simulink model "CCMAdcM"  (R2022b)
settings: Solver=FixedStepDiscrete  StartTime=0.0  StopTime=10.0  FixedStep=0.01  RelTol=1e-3
settings Model: Creator=blue.ge  LastModifiedBy=blue.ge
settings DataIOCC: SaveFormat=StructureWithTime
settings OptimizationCC: DefaultParameterBehavior=Inlined  EnhancedBackFolding=on  PassReuseOutputArgsAs=Individual arguments  ZeroExternalMemoryAtStartup=off  ZeroInternalMemoryAtStartup=off  OptimizeBlockOrder=speed  DifferentSizesBufferReuse=on  OptimizationCustomize=off  EfficientTunableParamExpr=on
settings DebuggingCC: ParameterTunabilityLossMsg=error
settings ModelReferenceCC: ModelReferenceNumInstancesAllowed=Single
settings SFSimCC: MATLABDynamicMemAlloc=off
settings RTWCC: Description=AUTOSAR  SystemTargetFile=autosar.tlc  GenCodeOnly=on  TemplateMakefile=ert_default_tmf
settings CodeAppCC: ForceParamTrailComments=on  IgnoreCustomStorageClasses=off  OperatorAnnotations=on  SimulinkDataObjDesc=on  SFDataObjDesc=on  InsertBlockDesc=on
settings STFCustomTargetCC: TargetLangStandard=C99 (ISO)  MultiwordLength=256  ParMdlRefBuildCompliant=on  CompOptLevelCompliant=on  IncludeMdlTerminateFcn=off  CombineOutputUpdateFcns=on  SuppressErrorStatus=on  ERTCustomFileBanners=on  SupportNonFinite=off  SupportComplex=off  SupportContinuousTime=off  SupportNonInlinedSFcns=off  RemoveResetFunc=on  MATLABClassNameForMDSCustomization=Simulink.SoftwareTarget.ERTCustomization  AutosarCompliant=on  UseToolchainInfoCompliant=on  LookupTableObjectStructAxisOrder=2,1,3,4,...  LUTObjectStructOrderEvenSpacing=Size,Table,Breakpoints  SystemTargetFile=autosar.tlc
settings ERTTargetCC: TargetLangStandard=C99 (ISO)  ConcurrentExecutionCompliant=off  IncludeMdlTerminateFcn=off  SuppressErrorStatus=on  SupportNonFinite=off  SupportComplex=off  ModelStepFunctionPrototypeControlCompliant=off  CPPClassGenCompliant=off  AutosarCompliant=on  LookupTableObjectStructAxisOrder=2,1,3,4,...  LUTObjectStructOrderEvenSpacing=Size,Table,Breakpoints  InlinedParameterPlacement=NonHierarchical  ERTCustomFileTemplate=example_file_process.tlc

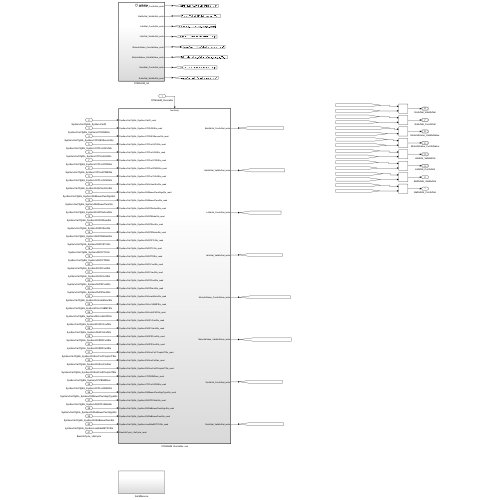
[thumbnail: Simulink's stored preview of the top-level diagram]
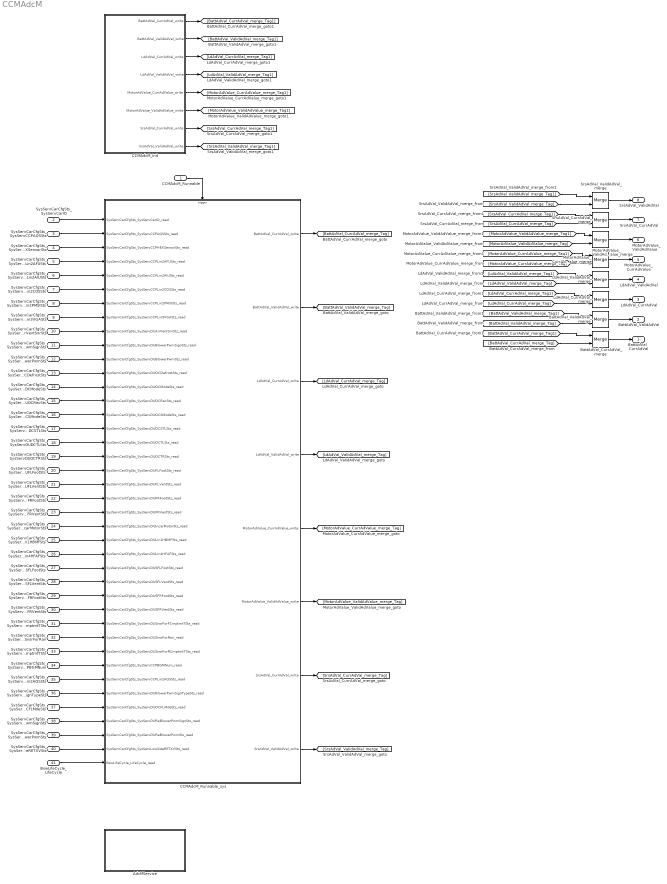
== system CCMAdcM ==
  CCMAdcM_Runnable : Inport  SampleTime=0.01  OutputFunctionCall=on
  SysServCarCfgSts_SysServCarID : Inport  Port=2  OutDataTypeStr=Enum: SysServCarID  PortDimensions=1
  SysServCarCfgSts_SysServCCPAQSSts : Inport  Port=3  OutDataTypeStr=UInt8  PortDimensions=1
  SysServCarCfgSts_SysServCCPHEXSensorSts : Inport  Port=4  OutDataTypeStr=UInt8  PortDimensions=1
  SysServCarCfgSts_SysServCCPLin2AFUSts : Inport  Port=5  OutDataTypeStr=Boolean  PortDimensions=1
  SysServCarCfgSts_SysServCCPLin2AIUSts : Inport  Port=6  OutDataTypeStr=UInt8  PortDimensions=1
  SysServCarCfgSts_SysServCCPLin2CDSSts : Inport  Port=7  OutDataTypeStr=Boolean  PortDimensions=1
  SysServCarCfgSts_SysServCCPLin2PMSISts : Inport  Port=8  OutDataTypeStr=Boolean  PortDimensions=1
  SysServCarCfgSts_SysServCCPLin3VGASts : Inport  Port=9  OutDataTypeStr=Boolean  PortDimensions=1
  SysServCarCfgSts_SysServDUAirVentSnrSts : Inport  Port=10  OutDataTypeStr=Boolean  PortDimensions=1
  SysServCarCfgSts_SysServDUBlowerPwmSignSts : Inport  Port=11  OutDataTypeStr=Boolean  PortDimensions=1
  SysServCarCfgSts_SysServDUBlowerPwmSts : Inport  Port=12  OutDataTypeStr=Boolean  PortDimensions=1
  SysServCarCfgSts_SysServDUDCDefrostSts : Inport  Port=13  OutDataTypeStr=UInt8  PortDimensions=1
  SysServCarCfgSts_SysServDUDCModeSts : Inport  Port=14  OutDataTypeStr=UInt8  PortDimensions=1
  SysServCarCfgSts_SysServDUDCRecSts : Inport  Port=15  OutDataTypeStr=UInt8  PortDimensions=1
  SysServCarCfgSts_SysServDUDCSModeSts : Inport  Port=16  OutDataTypeStr=UInt8  PortDimensions=1
  SysServCarCfgSts_SysServDUDCSTLSts : Inport  Port=17  OutDataTypeStr=UInt8  PortDimensions=1
  SysServCarCfgSts_SysServDUDCTLSts : Inport  Port=18  OutDataTypeStr=UInt8  PortDimensions=1
  SysServCarCfgSts_SysServDUDCTRSts : Inport  Port=19  OutDataTypeStr=UInt8  PortDimensions=1
  SysServCarCfgSts_SysServDUFLFootSts : Inport  Port=20  OutDataTypeStr=UInt8  PortDimensions=1
  SysServCarCfgSts_SysServDUFLVentSts : Inport  Port=21  OutDataTypeStr=UInt8  PortDimensions=1
  SysServCarCfgSts_SysServDUFRFootSts : Inport  Port=22  OutDataTypeStr=UInt8  PortDimensions=1
  SysServCarCfgSts_SysServDUFRVentSts : Inport  Port=23  OutDataTypeStr=UInt8  PortDimensions=1
  SysServCarCfgSts_SysServDUIncarMotorSts : Inport  Port=24  OutDataTypeStr=Boolean  PortDimensions=1
  SysServCarCfgSts_SysServDULin1HBMFSts : Inport  Port=25  OutDataTypeStr=Boolean  PortDimensions=1
  SysServCarCfgSts_SysServDULin4HFAFSts : Inport  Port=26  OutDataTypeStr=UInt32  PortDimensions=1
  SysServCarCfgSts_SysServDUSFLFootSts : Inport  Port=27  OutDataTypeStr=UInt8  PortDimensions=1
  SysServCarCfgSts_SysServDUSFLVentSts : Inport  Port=28  OutDataTypeStr=UInt8  PortDimensions=1
  SysServCarCfgSts_SysServDUSFRFootSts : Inport  Port=29  OutDataTypeStr=UInt8  PortDimensions=1
  SysServCarCfgSts_SysServDUSFRVentSts : Inport  Port=30  OutDataTypeStr=UInt8  PortDimensions=1
  SysServCarCfgSts_SysServDUSnsrForFCmptmtTSts : Inport  Port=31  OutDataTypeStr=UInt8  PortDimensions=1
  SysServCarCfgSts_SysServDUSnsrForRair : Inport  Port=32  OutDataTypeStr=UInt8  PortDimensions=1
  SysServCarCfgSts_SysServDUSnsrForRCmptmtTSts : Inport  Port=33  OutDataTypeStr=UInt8  PortDimensions=1
  SysServCarCfgSts_SysServCCPBGMNum : Inport  Port=34  OutDataTypeStr=UInt8  PortDimensions=1
  SysServCarCfgSts_SysServCCPLin2AQSSts : Inport  Port=35  OutDataTypeStr=Boolean  PortDimensions=1
  SysServCarCfgSts_SysServDUBlowerPwmSignTypeSts : Inport  Port=36  OutDataTypeStr=UInt8  PortDimensions=1
  SysServCarCfgSts_SysServDUDCFLMdeSts : Inport  Port=37  OutDataTypeStr=UInt8  PortDimensions=1
  SysServCarCfgSts_SysServDUReBlowerPwmSignSts : Inport  Port=38  OutDataTypeStr=UInt8  PortDimensions=1
  SysServCarCfgSts_SysServDUReBlowerPwmSts : Inport  Port=39  OutDataTypeStr=UInt8  PortDimensions=1
  SysServCarCfgSts_SysServLowSideRETXVSts : Inport  Port=40  OutDataTypeStr=UInt8  PortDimensions=1
  BswLifeCycle_LifeCycle : Inport  Port=41  OutDataTypeStr=Enum: CCMLifeCycleModeGroup  PortDimensions=1
  AdcMService : Subsystem
  BattAdVal_CurrAdVal_merge : Merge
  BattAdVal_ValidAdVal_merge : Merge
  CCMAdcM_Init : Subsystem
  CCMAdcM_Runnable_sys : Subsystem
  LdAdVal_CurrAdVal_merge : Merge
  LdAdVal_ValidAdVal_merge : Merge
  MotorAdValue_CurrAdValue_merge : Merge
  MotorAdValue_ValidAdValue_merge : Merge
  SrsAdVal_CurrAdVal_merge : Merge
  SrsAdVal_ValidAdVal_merge : Merge
  BattAdVal_CurrAdVal : Outport  OutDataTypeStr=BatCurrAd  PortDimensions=1
  BattAdVal_ValidAdVal : Outport  Port=2  OutDataTypeStr=BatValidAd  PortDimensions=1
  LdAdVal_CurrAdVal : Outport  Port=3  OutDataTypeStr=Bus: LdAd  PortDimensions=1  BusOutputAsStruct=on
  LdAdVal_ValidAdVal : Outport  Port=4  OutDataTypeStr=Bus: LdAd  PortDimensions=1  BusOutputAsStruct=on
  MotorAdValue_CurrAdValue : Outport  Port=5  OutDataTypeStr=Bus: MotorAdValueType  PortDimensions=1  BusOutputAsStruct=on
  MotorAdValue_ValidAdValue : Outport  Port=6  OutDataTypeStr=Bus: MotorAdValueType  PortDimensions=1  BusOutputAsStruct=on
  SrsAdVal_CurrAdVal : Outport  Port=7  OutDataTypeStr=Bus: SensorAd  PortDimensions=1  BusOutputAsStruct=on
  SrsAdVal_ValidAdVal : Outport  Port=8  OutDataTypeStr=Bus: SensorAd  PortDimensions=1  BusOutputAsStruct=on
  wires:
  SysServCarCfgSts_SysServCarID/1 -> CCMAdcM_Runnable_sys/1(SysServCarCfgSts_SysServCarID_read)
  SysServCarCfgSts_SysServCCPAQSSts/1 -> CCMAdcM_Runnable_sys/2(SysServCarCfgSts_SysServCCPAQSSts_read)
  SysServCarCfgSts_SysServCCPHEXSensorSts/1 -> CCMAdcM_Runnable_sys/3(SysServCarCfgSts_SysServCCPHEXSensorSts_read)
  SysServCarCfgSts_SysServCCPLin2AFUSts/1 -> CCMAdcM_Runnable_sys/4(SysServCarCfgSts_SysServCCPLin2AFUSts_read)
  SysServCarCfgSts_SysServCCPLin2AIUSts/1 -> CCMAdcM_Runnable_sys/5(SysServCarCfgSts_SysServCCPLin2AIUSts_read)
  SysServCarCfgSts_SysServCCPLin2CDSSts/1 -> CCMAdcM_Runnable_sys/6(SysServCarCfgSts_SysServCCPLin2CDSSts_read)
  SysServCarCfgSts_SysServCCPLin2PMSISts/1 -> CCMAdcM_Runnable_sys/7(SysServCarCfgSts_SysServCCPLin2PMSISts_read)
  SysServCarCfgSts_SysServCCPLin3VGASts/1 -> CCMAdcM_Runnable_sys/8(SysServCarCfgSts_SysServCCPLin3VGASts_read)
  SysServCarCfgSts_SysServDUAirVentSnrSts/1 -> CCMAdcM_Runnable_sys/9(SysServCarCfgSts_SysServDUAirVentSnrSts_read)
  SysServCarCfgSts_SysServDUBlowerPwmSignSts/1 -> CCMAdcM_Runnable_sys/10(SysServCarCfgSts_SysServDUBlowerPwmSignSts_read)
  SysServCarCfgSts_SysServDUBlowerPwmSts/1 -> CCMAdcM_Runnable_sys/11(SysServCarCfgSts_SysServDUBlowerPwmSts_read)
  SysServCarCfgSts_SysServDUDCDefrostSts/1 -> CCMAdcM_Runnable_sys/12(SysServCarCfgSts_SysServDUDCDefrostSts_read)
  SysServCarCfgSts_SysServDUDCModeSts/1 -> CCMAdcM_Runnable_sys/13(SysServCarCfgSts_SysServDUDCModeSts_read)
  SysServCarCfgSts_SysServDUDCRecSts/1 -> CCMAdcM_Runnable_sys/14(SysServCarCfgSts_SysServDUDCRecSts_read)
  SysServCarCfgSts_SysServDUDCSModeSts/1 -> CCMAdcM_Runnable_sys/15(SysServCarCfgSts_SysServDUDCSModeSts_read)
  SysServCarCfgSts_SysServDUDCSTLSts/1 -> CCMAdcM_Runnable_sys/16(SysServCarCfgSts_SysServDUDCSTLSts_read)
  SysServCarCfgSts_SysServDUDCTLSts/1 -> CCMAdcM_Runnable_sys/17(SysServCarCfgSts_SysServDUDCTLSts_read)
  SysServCarCfgSts_SysServDUDCTRSts/1 -> CCMAdcM_Runnable_sys/18(SysServCarCfgSts_SysServDUDCTRSts_read)
  SysServCarCfgSts_SysServDUFLFootSts/1 -> CCMAdcM_Runnable_sys/19(SysServCarCfgSts_SysServDUFLFootSts_read)
  SysServCarCfgSts_SysServDUFLVentSts/1 -> CCMAdcM_Runnable_sys/20(SysServCarCfgSts_SysServDUFLVentSts_read)
  SysServCarCfgSts_SysServDUFRFootSts/1 -> CCMAdcM_Runnable_sys/21(SysServCarCfgSts_SysServDUFRFootSts_read)
  SysServCarCfgSts_SysServDUFRVentSts/1 -> CCMAdcM_Runnable_sys/22(SysServCarCfgSts_SysServDUFRVentSts_read)
  SysServCarCfgSts_SysServDUIncarMotorSts/1 -> CCMAdcM_Runnable_sys/23(SysServCarCfgSts_SysServDUIncarMotorSts_read)
  SysServCarCfgSts_SysServDULin1HBMFSts/1 -> CCMAdcM_Runnable_sys/24(SysServCarCfgSts_SysServDULin1HBMFSts_read)
  SysServCarCfgSts_SysServDULin4HFAFSts/1 -> CCMAdcM_Runnable_sys/25(SysServCarCfgSts_SysServDULin4HFAFSts_read)
  SysServCarCfgSts_SysServDUSFLFootSts/1 -> CCMAdcM_Runnable_sys/26(SysServCarCfgSts_SysServDUSFLFootSts_read)
  SysServCarCfgSts_SysServDUSFLVentSts/1 -> CCMAdcM_Runnable_sys/27(SysServCarCfgSts_SysServDUSFLVentSts_read)
  SysServCarCfgSts_SysServDUSFRFootSts/1 -> CCMAdcM_Runnable_sys/28(SysServCarCfgSts_SysServDUSFRFootSts_read)
  SysServCarCfgSts_SysServDUSFRVentSts/1 -> CCMAdcM_Runnable_sys/29(SysServCarCfgSts_SysServDUSFRVentSts_read)
  SysServCarCfgSts_SysServDUSnsrForFCmptmtTSts/1 -> CCMAdcM_Runnable_sys/30(SysServCarCfgSts_SysServDUSnsrForFCmptmtTSts_read)
  SysServCarCfgSts_SysServDUSnsrForRair/1 -> CCMAdcM_Runnable_sys/31(SysServCarCfgSts_SysServDUSnsrForRair_read)
  SysServCarCfgSts_SysServDUSnsrForRCmptmtTSts/1 -> CCMAdcM_Runnable_sys/32(SysServCarCfgSts_SysServDUSnsrForRCmptmtTSts_read)
  SysServCarCfgSts_SysServCCPBGMNum/1 -> CCMAdcM_Runnable_sys/33(SysServCarCfgSts_SysServCCPBGMNum_read)
  SysServCarCfgSts_SysServCCPLin2AQSSts/1 -> CCMAdcM_Runnable_sys/34(SysServCarCfgSts_SysServCCPLin2AQSSts_read)
  SysServCarCfgSts_SysServDUBlowerPwmSignTypeSts/1 -> CCMAdcM_Runnable_sys/35(SysServCarCfgSts_SysServDUBlowerPwmSignTypeSts_read)
  SysServCarCfgSts_SysServDUDCFLMdeSts/1 -> CCMAdcM_Runnable_sys/36(SysServCarCfgSts_SysServDUDCFLMdeSts_read)
  SysServCarCfgSts_SysServDUReBlowerPwmSignSts/1 -> CCMAdcM_Runnable_sys/37(SysServCarCfgSts_SysServDUReBlowerPwmSignSts_read)
  SysServCarCfgSts_SysServDUReBlowerPwmSts/1 -> CCMAdcM_Runnable_sys/38(SysServCarCfgSts_SysServDUReBlowerPwmSts_read)
  SysServCarCfgSts_SysServLowSideRETXVSts/1 -> CCMAdcM_Runnable_sys/39(SysServCarCfgSts_SysServLowSideRETXVSts_read)
  BattAdVal_CurrAdVal_merge/1 -> BattAdVal_CurrAdVal/1
  BattAdVal_ValidAdVal_merge/1 -> BattAdVal_ValidAdVal/1
  LdAdVal_CurrAdVal_merge/1 -> LdAdVal_CurrAdVal/1
  LdAdVal_ValidAdVal_merge/1 -> LdAdVal_ValidAdVal/1
  MotorAdValue_CurrAdValue_merge/1 -> MotorAdValue_CurrAdValue/1
  MotorAdValue_ValidAdValue_merge/1 -> MotorAdValue_ValidAdValue/1
  SrsAdVal_CurrAdVal_merge/1 -> SrsAdVal_CurrAdVal/1
  SrsAdVal_ValidAdVal_merge/1 -> SrsAdVal_ValidAdVal/1
  BswLifeCycle_LifeCycle/1 -> CCMAdcM_Runnable_sys/40(BswLifeCycle_LifeCycle_read)
  CCMAdcM_Runnable_sys/8(SrsAdVal_ValidAdVal_write) -> [SrsAdVal_ValidAdVal_merge_Tag]
  [SrsAdVal_ValidAdVal_merge_Tag] -> SrsAdVal_ValidAdVal_merge/2
  CCMAdcM_Init/8(SrsAdVal_ValidAdVal_write) -> [SrsAdVal_ValidAdVal_merge_Tag1]
  [SrsAdVal_ValidAdVal_merge_Tag1] -> SrsAdVal_ValidAdVal_merge/1
  CCMAdcM_Runnable_sys/7(SrsAdVal_CurrAdVal_write) -> [SrsAdVal_CurrAdVal_merge_Tag]
  [SrsAdVal_CurrAdVal_merge_Tag] -> SrsAdVal_CurrAdVal_merge/2
  CCMAdcM_Init/7(SrsAdVal_CurrAdVal_write) -> [SrsAdVal_CurrAdVal_merge_Tag1]
  [SrsAdVal_CurrAdVal_merge_Tag1] -> SrsAdVal_CurrAdVal_merge/1
  CCMAdcM_Runnable_sys/6(MotorAdValue_ValidAdValue_write) -> [MotorAdValue_ValidAdValue_merge_Tag]
  [MotorAdValue_ValidAdValue_merge_Tag] -> MotorAdValue_ValidAdValue_merge/2
  CCMAdcM_Init/6(MotorAdValue_ValidAdValue_write) -> [MotorAdValue_ValidAdValue_merge_Tag1]
  [MotorAdValue_ValidAdValue_merge_Tag1] -> MotorAdValue_ValidAdValue_merge/1
  CCMAdcM_Runnable_sys/5(MotorAdValue_CurrAdValue_write) -> [MotorAdValue_CurrAdValue_merge_Tag]
  [MotorAdValue_CurrAdValue_merge_Tag] -> MotorAdValue_CurrAdValue_merge/2
  CCMAdcM_Init/5(MotorAdValue_CurrAdValue_write) -> [MotorAdValue_CurrAdValue_merge_Tag1]
  [MotorAdValue_CurrAdValue_merge_Tag1] -> MotorAdValue_CurrAdValue_merge/1
  CCMAdcM_Runnable_sys/4(LdAdVal_ValidAdVal_write) -> [LdAdVal_ValidAdVal_merge_Tag]
  [LdAdVal_ValidAdVal_merge_Tag] -> LdAdVal_ValidAdVal_merge/2
  CCMAdcM_Init/4(LdAdVal_ValidAdVal_write) -> [LdAdVal_ValidAdVal_merge_Tag1]
  [LdAdVal_ValidAdVal_merge_Tag1] -> LdAdVal_ValidAdVal_merge/1
  CCMAdcM_Runnable_sys/3(LdAdVal_CurrAdVal_write) -> [LdAdVal_CurrAdVal_merge_Tag]
  [LdAdVal_CurrAdVal_merge_Tag] -> LdAdVal_CurrAdVal_merge/2
  CCMAdcM_Init/3(LdAdVal_CurrAdVal_write) -> [LdAdVal_CurrAdVal_merge_Tag1]
  [LdAdVal_CurrAdVal_merge_Tag1] -> LdAdVal_CurrAdVal_merge/1
  CCMAdcM_Runnable_sys/2(BattAdVal_ValidAdVal_write) -> [BattAdVal_ValidAdVal_merge_Tag]
  [BattAdVal_ValidAdVal_merge_Tag] -> BattAdVal_ValidAdVal_merge/2
  CCMAdcM_Init/2(BattAdVal_ValidAdVal_write) -> [BattAdVal_ValidAdVal_merge_Tag1]
  [BattAdVal_ValidAdVal_merge_Tag1] -> BattAdVal_ValidAdVal_merge/1
  CCMAdcM_Runnable_sys/1(BattAdVal_CurrAdVal_write) -> [BattAdVal_CurrAdVal_merge_Tag]
  [BattAdVal_CurrAdVal_merge_Tag] -> BattAdVal_CurrAdVal_merge/2
  CCMAdcM_Init/1(BattAdVal_CurrAdVal_write) -> [BattAdVal_CurrAdVal_merge_Tag1]
  [BattAdVal_CurrAdVal_merge_Tag1] -> BattAdVal_CurrAdVal_merge/1
  CCMAdcM_Runnable/1 -> CCMAdcM_Runnable_sys/Trigger

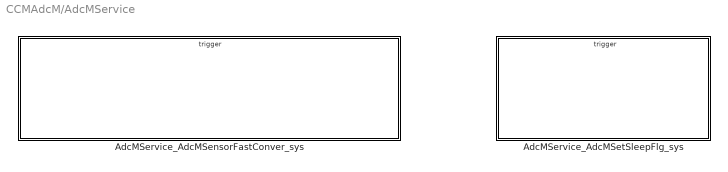
== system CCMAdcM/AdcMService ==
  AdcMService_AdcMSensorFastConver_sys : Subsystem
  AdcMService_AdcMSetSleepFlg_sys : Subsystem

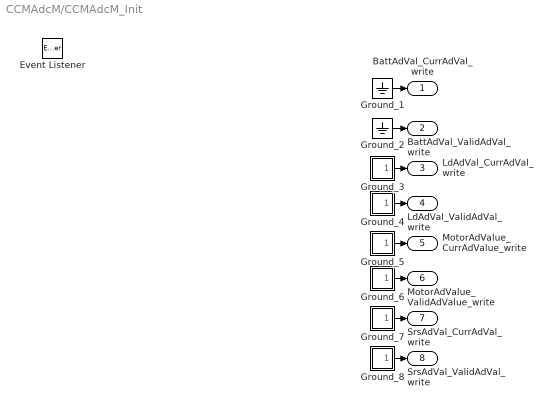
== system CCMAdcM/CCMAdcM_Init ==
  Event Listener : EventListener  EventName=reset
  Ground_1 : Ground
  Ground_2 : Ground
  Ground_3 : Subsystem
  Ground_4 : Subsystem
  Ground_5 : Subsystem
  Ground_6 : Subsystem
  Ground_7 : Subsystem
  Ground_8 : Subsystem
  BattAdVal_CurrAdVal_write : Outport
  BattAdVal_ValidAdVal_write : Outport  Port=2
  LdAdVal_CurrAdVal_write : Outport  Port=3
  LdAdVal_ValidAdVal_write : Outport  Port=4
  MotorAdValue_CurrAdValue_write : Outport  Port=5
  MotorAdValue_ValidAdValue_write : Outport  Port=6
  SrsAdVal_CurrAdVal_write : Outport  Port=7
  SrsAdVal_ValidAdVal_write : Outport  Port=8
  wires:
  Ground_1/1 -> BattAdVal_CurrAdVal_write/1
  Ground_2/1 -> BattAdVal_ValidAdVal_write/1
  Ground_3/1(Out1) -> LdAdVal_CurrAdVal_write/1
  Ground_4/1(Out1) -> LdAdVal_ValidAdVal_write/1
  Ground_5/1(Out1) -> MotorAdValue_CurrAdValue_write/1
  Ground_6/1(Out1) -> MotorAdValue_ValidAdValue_write/1
  Ground_7/1(Out1) -> SrsAdVal_CurrAdVal_write/1
  Ground_8/1(Out1) -> SrsAdVal_ValidAdVal_write/1

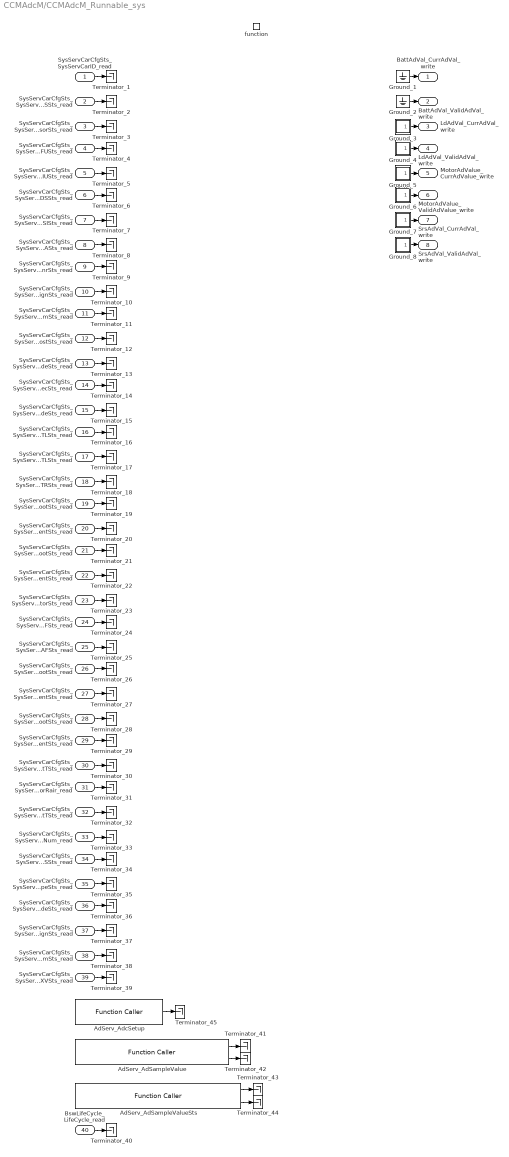
== system CCMAdcM/CCMAdcM_Runnable_sys ==
  SysServCarCfgSts_SysServCarID_read : Inport
  SysServCarCfgSts_SysServCCPAQSSts_read : Inport  Port=2
  SysServCarCfgSts_SysServCCPHEXSensorSts_read : Inport  Port=3
  SysServCarCfgSts_SysServCCPLin2AFUSts_read : Inport  Port=4
  SysServCarCfgSts_SysServCCPLin2AIUSts_read : Inport  Port=5
  SysServCarCfgSts_SysServCCPLin2CDSSts_read : Inport  Port=6
  SysServCarCfgSts_SysServCCPLin2PMSISts_read : Inport  Port=7
  SysServCarCfgSts_SysServCCPLin3VGASts_read : Inport  Port=8
  SysServCarCfgSts_SysServDUAirVentSnrSts_read : Inport  Port=9
  SysServCarCfgSts_SysServDUBlowerPwmSignSts_read : Inport  Port=10
  SysServCarCfgSts_SysServDUBlowerPwmSts_read : Inport  Port=11
  SysServCarCfgSts_SysServDUDCDefrostSts_read : Inport  Port=12
  SysServCarCfgSts_SysServDUDCModeSts_read : Inport  Port=13
  SysServCarCfgSts_SysServDUDCRecSts_read : Inport  Port=14
  SysServCarCfgSts_SysServDUDCSModeSts_read : Inport  Port=15
  SysServCarCfgSts_SysServDUDCSTLSts_read : Inport  Port=16
  SysServCarCfgSts_SysServDUDCTLSts_read : Inport  Port=17
  SysServCarCfgSts_SysServDUDCTRSts_read : Inport  Port=18
  SysServCarCfgSts_SysServDUFLFootSts_read : Inport  Port=19
  SysServCarCfgSts_SysServDUFLVentSts_read : Inport  Port=20
  SysServCarCfgSts_SysServDUFRFootSts_read : Inport  Port=21
  SysServCarCfgSts_SysServDUFRVentSts_read : Inport  Port=22
  SysServCarCfgSts_SysServDUIncarMotorSts_read : Inport  Port=23
  SysServCarCfgSts_SysServDULin1HBMFSts_read : Inport  Port=24
  SysServCarCfgSts_SysServDULin4HFAFSts_read : Inport  Port=25
  SysServCarCfgSts_SysServDUSFLFootSts_read : Inport  Port=26
  SysServCarCfgSts_SysServDUSFLVentSts_read : Inport  Port=27
  SysServCarCfgSts_SysServDUSFRFootSts_read : Inport  Port=28
  SysServCarCfgSts_SysServDUSFRVentSts_read : Inport  Port=29
  SysServCarCfgSts_SysServDUSnsrForFCmptmtTSts_read : Inport  Port=30
  SysServCarCfgSts_SysServDUSnsrForRair_read : Inport  Port=31
  SysServCarCfgSts_SysServDUSnsrForRCmptmtTSts_read : Inport  Port=32
  SysServCarCfgSts_SysServCCPBGMNum_read : Inport  Port=33
  SysServCarCfgSts_SysServCCPLin2AQSSts_read : Inport  Port=34
  SysServCarCfgSts_SysServDUBlowerPwmSignTypeSts_read : Inport  Port=35
  SysServCarCfgSts_SysServDUDCFLMdeSts_read : Inport  Port=36
  SysServCarCfgSts_SysServDUReBlowerPwmSignSts_read : Inport  Port=37
  SysServCarCfgSts_SysServDUReBlowerPwmSts_read : Inport  Port=38
  SysServCarCfgSts_SysServLowSideRETXVSts_read : Inport  Port=39
  BswLifeCycle_LifeCycle_read : Inport  Port=40
  function : TriggerPort  TriggerType=function-call  VariantControl=(inherit)  SampleTimeType=periodic  SampleTime=-1
  AdServ_AdSampleValue : FunctionCaller  FunctionPrototype=[AdValue,ERR] = AdServ_AdSampleValue()  OutputArgumentSpecifications=P_SampleValueType, P_Std_ReturnType
  AdServ_AdSampleValueSts : FunctionCaller  FunctionPrototype=[AdValue,ERR] = AdServ_AdSampleValueSts()  OutputArgumentSpecifications=P_SampleValueTypeSts, P_Std_ReturnType
  AdServ_AdcSetup : FunctionCaller  FunctionPrototype=ERR = AdServ_AdcSetup()  OutputArgumentSpecifications=P_Std_ReturnType
  Ground_1 : Ground
  Ground_2 : Ground
  Ground_3 : Subsystem
  Ground_4 : Subsystem
  Ground_5 : Subsystem
  Ground_6 : Subsystem
  Ground_7 : Subsystem
  Ground_8 : Subsystem
  Terminator_{1..45} (45 alike) : Terminator
  BattAdVal_CurrAdVal_write : Outport
      settings: EnsureOutportIsVirtual=on
  BattAdVal_ValidAdVal_write : Outport  Port=2
      settings: EnsureOutportIsVirtual=on
  LdAdVal_CurrAdVal_write : Outport  Port=3
      settings: EnsureOutportIsVirtual=on
  LdAdVal_ValidAdVal_write : Outport  Port=4
      settings: EnsureOutportIsVirtual=on
  MotorAdValue_CurrAdValue_write : Outport  Port=5
      settings: EnsureOutportIsVirtual=on
  MotorAdValue_ValidAdValue_write : Outport  Port=6
      settings: EnsureOutportIsVirtual=on
  SrsAdVal_CurrAdVal_write : Outport  Port=7
      settings: EnsureOutportIsVirtual=on
  SrsAdVal_ValidAdVal_write : Outport  Port=8
      settings: EnsureOutportIsVirtual=on
  wires:
  SysServCarCfgSts_SysServCarID_read/1 -> Terminator_1/1
  SysServCarCfgSts_SysServCCPAQSSts_read/1 -> Terminator_2/1
  SysServCarCfgSts_SysServCCPHEXSensorSts_read/1 -> Terminator_3/1
  SysServCarCfgSts_SysServCCPLin2AFUSts_read/1 -> Terminator_4/1
  SysServCarCfgSts_SysServCCPLin2AIUSts_read/1 -> Terminator_5/1
  SysServCarCfgSts_SysServCCPLin2CDSSts_read/1 -> Terminator_6/1
  SysServCarCfgSts_SysServCCPLin2PMSISts_read/1 -> Terminator_7/1
  SysServCarCfgSts_SysServCCPLin3VGASts_read/1 -> Terminator_8/1
  SysServCarCfgSts_SysServDUAirVentSnrSts_read/1 -> Terminator_9/1
  SysServCarCfgSts_SysServDUBlowerPwmSignSts_read/1 -> Terminator_10/1
  SysServCarCfgSts_SysServDUBlowerPwmSts_read/1 -> Terminator_11/1
  SysServCarCfgSts_SysServDUDCDefrostSts_read/1 -> Terminator_12/1
  SysServCarCfgSts_SysServDUDCModeSts_read/1 -> Terminator_13/1
  SysServCarCfgSts_SysServDUDCRecSts_read/1 -> Terminator_14/1
  SysServCarCfgSts_SysServDUDCSModeSts_read/1 -> Terminator_15/1
  SysServCarCfgSts_SysServDUDCSTLSts_read/1 -> Terminator_16/1
  SysServCarCfgSts_SysServDUDCTLSts_read/1 -> Terminator_17/1
  SysServCarCfgSts_SysServDUDCTRSts_read/1 -> Terminator_18/1
  SysServCarCfgSts_SysServDUFLFootSts_read/1 -> Terminator_19/1
  SysServCarCfgSts_SysServDUFLVentSts_read/1 -> Terminator_20/1
  SysServCarCfgSts_SysServDUFRFootSts_read/1 -> Terminator_21/1
  SysServCarCfgSts_SysServDUFRVentSts_read/1 -> Terminator_22/1
  SysServCarCfgSts_SysServDUIncarMotorSts_read/1 -> Terminator_23/1
  SysServCarCfgSts_SysServDULin1HBMFSts_read/1 -> Terminator_24/1
  SysServCarCfgSts_SysServDULin4HFAFSts_read/1 -> Terminator_25/1
  SysServCarCfgSts_SysServDUSFLFootSts_read/1 -> Terminator_26/1
  SysServCarCfgSts_SysServDUSFLVentSts_read/1 -> Terminator_27/1
  SysServCarCfgSts_SysServDUSFRFootSts_read/1 -> Terminator_28/1
  SysServCarCfgSts_SysServDUSFRVentSts_read/1 -> Terminator_29/1
  SysServCarCfgSts_SysServDUSnsrForFCmptmtTSts_read/1 -> Terminator_30/1
  SysServCarCfgSts_SysServDUSnsrForRair_read/1 -> Terminator_31/1
  SysServCarCfgSts_SysServDUSnsrForRCmptmtTSts_read/1 -> Terminator_32/1
  SysServCarCfgSts_SysServCCPBGMNum_read/1 -> Terminator_33/1
  SysServCarCfgSts_SysServCCPLin2AQSSts_read/1 -> Terminator_34/1
  SysServCarCfgSts_SysServDUBlowerPwmSignTypeSts_read/1 -> Terminator_35/1
  SysServCarCfgSts_SysServDUDCFLMdeSts_read/1 -> Terminator_36/1
  SysServCarCfgSts_SysServDUReBlowerPwmSignSts_read/1 -> Terminator_37/1
  SysServCarCfgSts_SysServDUReBlowerPwmSts_read/1 -> Terminator_38/1
  SysServCarCfgSts_SysServLowSideRETXVSts_read/1 -> Terminator_39/1
  BswLifeCycle_LifeCycle_read/1 -> Terminator_40/1
  AdServ_AdSampleValue/1 -> Terminator_41/1
  AdServ_AdSampleValue/2 -> Terminator_42/1
  AdServ_AdSampleValueSts/1 -> Terminator_43/1
  AdServ_AdSampleValueSts/2 -> Terminator_44/1
  AdServ_AdcSetup/1 -> Terminator_45/1
  Ground_1/1 -> BattAdVal_CurrAdVal_write/1
  Ground_2/1 -> BattAdVal_ValidAdVal_write/1
  Ground_3/1(Out1) -> LdAdVal_CurrAdVal_write/1
  Ground_4/1(Out1) -> LdAdVal_ValidAdVal_write/1
  Ground_5/1(Out1) -> MotorAdValue_CurrAdValue_write/1
  Ground_6/1(Out1) -> MotorAdValue_ValidAdValue_write/1
  Ground_7/1(Out1) -> SrsAdVal_CurrAdVal_write/1
  Ground_8/1(Out1) -> SrsAdVal_ValidAdVal_write/1

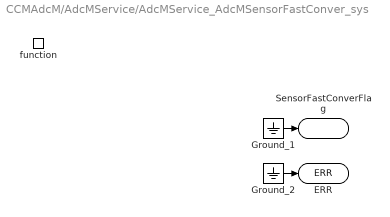
== system CCMAdcM/AdcMService/AdcMService_AdcMSensorFastConver_sys ==
  function : TriggerPort  TriggerType=function-call  IsSimulinkFunction=on  FunctionName=AdcMService_AdcMSensorFastConver  VariantControl=(inherit)
  Ground_1 : Ground
  Ground_2 : Ground
  SensorFastConverFlag : ArgOut  OutDataTypeStr=AdcMSensorFastConver  ArgumentName=SensorFastConverFlag
  ERR : ArgOut  Port=2  OutDataTypeStr=Std_ReturnType  ArgumentName=ERR
  wires:
  Ground_1/1 -> SensorFastConverFlag/1
  Ground_2/1 -> ERR/1

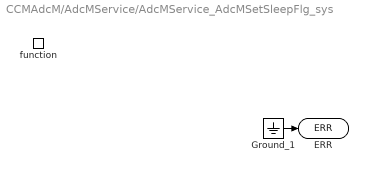
== system CCMAdcM/AdcMService/AdcMService_AdcMSetSleepFlg_sys ==
  function : TriggerPort  TriggerType=function-call  IsSimulinkFunction=on  FunctionName=AdcMService_AdcMSetSleepFlg  VariantControl=(inherit)
  Ground_1 : Ground
  ERR : ArgOut  OutDataTypeStr=Std_ReturnType  ArgumentName=ERR
  wires:
  Ground_1/1 -> ERR/1

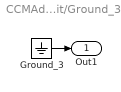
== system CCMAdcM/CCMAdcM_Init/Ground_3 ==
  Ground_3 : Ground
  Out1 : Outport  OutDataTypeStr=Bus: LdAd  PortDimensions=1
  wires:
  Ground_3/1 -> Out1/1

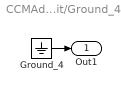
== system CCMAdcM/CCMAdcM_Init/Ground_4 ==
  Ground_4 : Ground
  Out1 : Outport  OutDataTypeStr=Bus: LdAd  PortDimensions=1
  wires:
  Ground_4/1 -> Out1/1

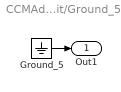
== system CCMAdcM/CCMAdcM_Init/Ground_5 ==
  Ground_5 : Ground
  Out1 : Outport  OutDataTypeStr=Bus: MotorAdValueType  PortDimensions=1
  wires:
  Ground_5/1 -> Out1/1

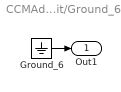
== system CCMAdcM/CCMAdcM_Init/Ground_6 ==
  Ground_6 : Ground
  Out1 : Outport  OutDataTypeStr=Bus: MotorAdValueType  PortDimensions=1
  wires:
  Ground_6/1 -> Out1/1

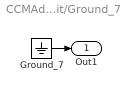
== system CCMAdcM/CCMAdcM_Init/Ground_7 ==
  Ground_7 : Ground
  Out1 : Outport  OutDataTypeStr=Bus: SensorAd  PortDimensions=1
  wires:
  Ground_7/1 -> Out1/1

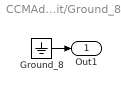
== system CCMAdcM/CCMAdcM_Init/Ground_8 ==
  Ground_8 : Ground
  Out1 : Outport  OutDataTypeStr=Bus: SensorAd  PortDimensions=1
  wires:
  Ground_8/1 -> Out1/1

== system CCMAdcM/CCMAdcM_Runnable_sys/Ground_3 ==
  Ground_3 : Ground
  Out1 : Outport  OutDataTypeStr=Bus: LdAd  PortDimensions=1
  wires:
  Ground_3/1 -> Out1/1

== system CCMAdcM/CCMAdcM_Runnable_sys/Ground_4 ==
  Ground_4 : Ground
  Out1 : Outport  OutDataTypeStr=Bus: LdAd  PortDimensions=1
  wires:
  Ground_4/1 -> Out1/1

== system CCMAdcM/CCMAdcM_Runnable_sys/Ground_5 ==
  Ground_5 : Ground
  Out1 : Outport  OutDataTypeStr=Bus: MotorAdValueType  PortDimensions=1
  wires:
  Ground_5/1 -> Out1/1

== system CCMAdcM/CCMAdcM_Runnable_sys/Ground_6 ==
  Ground_6 : Ground
  Out1 : Outport  OutDataTypeStr=Bus: MotorAdValueType  PortDimensions=1
  wires:
  Ground_6/1 -> Out1/1

== system CCMAdcM/CCMAdcM_Runnable_sys/Ground_7 ==
  Ground_7 : Ground
  Out1 : Outport  OutDataTypeStr=Bus: SensorAd  PortDimensions=1
  wires:
  Ground_7/1 -> Out1/1

== system CCMAdcM/CCMAdcM_Runnable_sys/Ground_8 ==
  Ground_8 : Ground
  Out1 : Outport  OutDataTypeStr=Bus: SensorAd  PortDimensions=1
  wires:
  Ground_8/1 -> Out1/1
